simulink model "FlightSim"  (R2023a) (continued)
== system FlightSim/Aerodyanmic Forces/Axial Drag == (continued)
  bodyLength/1 -> CD(friction) calculation/1(bodyLength)
  finArea/1 -> CD(friction) calculation/2(finPlanformArea)
  finMACLength/1 -> CD(friction) calculation/3(MACLength)
  finThickness/1 -> CD(friction) calculation/6(finThickness), Body Pressure Drag/2(finThicknes)
  finCount/1 -> allFinCdFriciton/2, Body Pressure Drag/3(finCount)
  finProfileType/1 -> Body Pressure Drag/4(finProfileType)
  finSpan/1 -> Body Pressure Drag/5(finSpan)
  noseConeType/1 -> Body Pressure Drag/7(noseConeType)
  noseConeWettedArea/1 -> CD(friction) calculation/7(noseConeWettedArea)
  noseConeJoinAngle/1 -> Body Pressure Drag/8(noseConeJoinAngle)
  launchLugLength/1 -> Body Pressure Drag/9(launchLugLength)
  launchLugOuterDiameter/1 -> Body Pressure Drag/10(launchLugOuterDiameter)
  launchLugInnerDiameter/1 -> Body Pressure Drag/11(launchLugInnerDiameter)
  machNum/1 -> Body Pressure Drag/12(MachNum), SkinFrictionCoefficient/5(MachNum)
  AOA/1 -> CDtoAxailCD/2(AOA)
  finFrontSweepAngle/1 -> Body Pressure Drag/6(finFrontSweepAngle)
  SkinFrictionCoefficient/1(Cf) -> Display/1, CD(friction) calculation/4(Cf)
  rocketLength/1 -> SkinFrictionCoefficient/1(rocketLength)
  rocketVelocity/1 -> SkinFrictionCoefficient/2(rocketVelocity)
  airKinematicViscocity/1 -> SkinFrictionCoefficient/3(kinematic Viscosity)
  bodyR_S (roughness)/1 -> SkinFrictionCoefficient/4(R_s)
  CD(friction) calculation/3(Cd Friction Single Fin) -> allFinCdFriciton/1
  allFinCdFriciton/1 -> Add1/3, Display3/1
  noseConeHeight/1 -> CD(friction) calculation/8(noseConeHeight)
  CD(friction) calculation/2(Cd Friction Nosecone) -> Display1/1, Add1/2
  Add1/1 -> totalFrictionCD/1, Add/1

== system FlightSim/Aerodyanmic Forces/Canard Roll Calc ==
  note: FinSetCalc.Java file,line 184
  bodyDiameter : Inport
  finRootChord : Inport  Port=2
  finTailChord : Inport  Port=3
  finSpan : Inport  Port=4
  radialMACPosition : Inport  Port=5
  finCount : Inport  Port=6
  singleFinCNa : Inport  Port=7
  finCantAngle : Inport  Port=8
  " MachNum" : Inport  Port=9
  " rollRate" : Inport  Port=10
  " rocketVelocity" : Inport  Port=11
  Display : Display
  Display1 : Display
  Display2 : Display
  Product : Product
  RollDampeningCoefficient : Subsystem
  RollForcingCoefficient : Subsystem
  Subtract : Sum  Inputs=+-
  cRoll : Outport
  wires:
  bodyDiameter/1 -> RollDampeningCoefficient/3(bodyDiameter), RollForcingCoefficient/1(bodyDiameter)
  finRootChord/1 -> RollDampeningCoefficient/1(rootChord)
  finSpan/1 -> RollDampeningCoefficient/4(span), RollForcingCoefficient/5(finSpan)
  finCantAngle/1 -> RollForcingCoefficient/4(finCantAngle)
  radialMACPosition/1 -> RollForcingCoefficient/2(radialMACPosition)
  finTailChord/1 -> RollDampeningCoefficient/2(tailChord)
  finCount/1 -> Product/1
  singleFinCNa/1 -> RollForcingCoefficient/3(CNa)
  RollForcingCoefficient/1(singeFinRollForcing) -> Display/1, Subtract/1
  RollDampeningCoefficient/1(singleFinRollDamping) -> Display1/1, Subtract/2
  " MachNum"/1 -> RollDampeningCoefficient/5(MachNum)
  " rollRate"/1 -> RollDampeningCoefficient/6(rollRate)
  " rocketVelocity"/1 -> RollDampeningCoefficient/7(rocketVelocity)
  Subtract/1 -> Display2/1, Product/2
  Product/1 -> cRoll/1

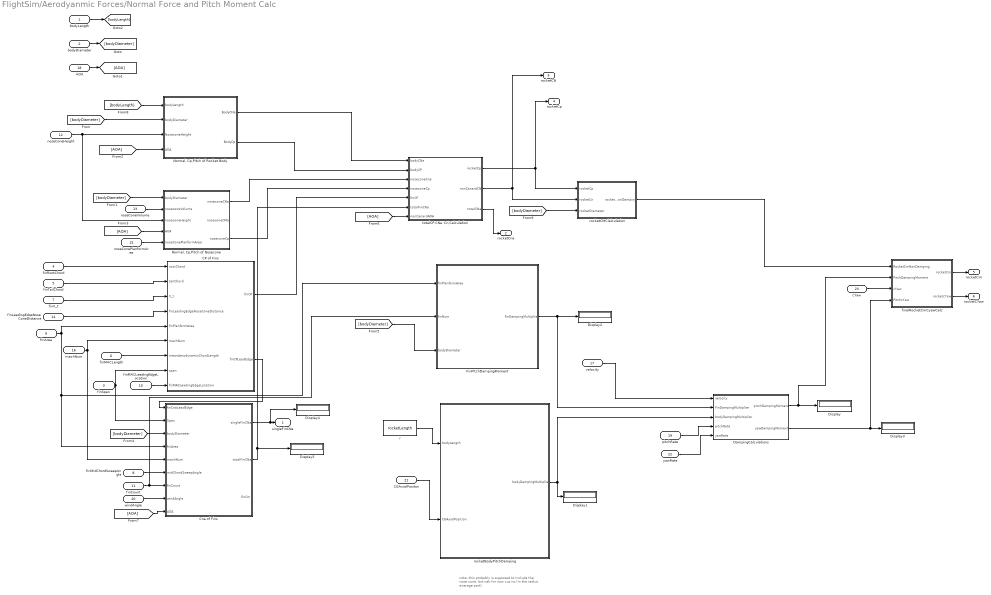
== system FlightSim/Aerodyanmic Forces/Normal Force and Pitch Moment Calc ==
  note: note: this probably is supposed to include the nose cone, but nah for now cuz no (in the radius average part)
  bodyLength : Inport
  bodyDiameter : Inport  Port=2
  finSpan : Inport  Port=3
  finRootChord : Inport  Port=4
  finTailChord : Inport  Port=5
  finMACLength : Inport  Port=6
  finX_t : Inport  Port=7
  finMidChordSweepAngle : Inport  Port=8
  finArea : Inport  Port=9
  finMACLeadingEdgeLocation : Inport  Port=10
  finCount : Inport  Port=11
  noseConeHeight : Inport  Port=12
  noseConeVolume : Inport  Port=13
  finLeadingEdgeNoseConeDistance : Inport  Port=14
  noseConePlanformArea : Inport  Port=15
  machNum : Inport  Port=16
  velocity : Inport  Port=17
  AOA : Inport  Port=18
  pitchRate : Inport  Port=19
  windAngle : Inport  Port=20
  CGAxialPositon : Inport  Port=21
  yawRate : Inport  Port=22
  cYaw : Inport  Port=23
  CP of Fins : Subsystem
  Cna of Fins : Subsystem
  DampingCalculations : Subsystem
  Display{,1..5} (6 alike) : Display
  FinPitchDampingMoment : Subsystem
  "Normal, Cp,Pitch of Nosecone" : Subsystem
  "Normal, Cp,Pitch of Rocket Body" : Subsystem
  finalRocketCmCyawCalc : Subsystem
  r : Constant  Value=rocketLength
  rocketBodyPitchDamping : Subsystem
  rocketCMClalculation : Subsystem
  "totalCP,CNa, Cn,Calculation" : Subsystem
  singleFinCNa : Outport
  rocketCna : Outport  Port=2
  rocketCN : Outport  Port=3
  rocketCp : Outport  Port=4
  rocketCm : Outport  Port=5
  rocketCYaw : Outport  Port=6
  wires:
  "Normal, Cp,Pitch of Rocket Body"/1(BodyCNa) -> "totalCP,CNa, Cn,Calculation"/1(bodyCNa)
  "Normal, Cp,Pitch of Rocket Body"/2(BodyCp) -> "totalCP,CNa, Cn,Calculation"/2(bodyCP)
  CP of Fins/1(finCP) -> "totalCP,CNa, Cn,Calculation"/5(finCP)
  "Normal, Cp,Pitch of Nosecone"/3(noseconeCp) -> "totalCP,CNa, Cn,Calculation"/4(noseconeCp)
  "Normal, Cp,Pitch of Nosecone"/1(noseconeCNa) -> "totalCP,CNa, Cn,Calculation"/3(noseconeCna)
  "totalCP,CNa, Cn,Calculation"/3(totalCNa) -> rocketCna/1
  "totalCP,CNa, Cn,Calculation"/1(rocketCp) -> rocketCMClalculation/1(rocketCp), rocketCp/1
  bodyDiameter/1 -> [bodyDiameter]
  [bodyDiameter] -> "Normal, Cp,Pitch of Rocket Body"/2(bodyDiameter)
  noseConeHeight/1 -> "Normal, Cp,Pitch of Rocket Body"/3(NoseconeHeight), "Normal, Cp,Pitch of Nosecone"/3(noseconeHeight)
  [bodyDiameter] -> "Normal, Cp,Pitch of Nosecone"/1(bodyDiameter)
  noseConeVolume/1 -> "Normal, Cp,Pitch of Nosecone"/2(noseconeVolume)
  AOA/1 -> [AOA]
  [AOA] -> "Normal, Cp,Pitch of Rocket Body"/4(AOA)
  [AOA] -> "Normal, Cp,Pitch of Nosecone"/4(AOA)
  finRootChord/1 -> CP of Fins/1(rootChord)
  finTailChord/1 -> CP of Fins/2(tailChord)
  finX_t/1 -> CP of Fins/3(X_t)
  finSpan/1 -> CP of Fins/8(span), Cna of Fins/2(Span)
  finMACLength/1 -> CP of Fins/7(meanAerodynamicChordLength)
  [bodyDiameter] -> Cna of Fins/3(bodyDiameter)
  finArea/1 -> CP of Fins/5(finPlanformArea), FinPitchDampingMoment/1(finPlanformArea), Cna of Fins/4(finArea)
  machNum/1 -> CP of Fins/6(machNum), Cna of Fins/5(machNum)
  finMidChordSweepAngle/1 -> Cna of Fins/6(midChordSweepAngle)
  finCount/1 -> Cna of Fins/7(finCount), FinPitchDampingMoment/2(finNum)
  finLeadingEdgeNoseConeDistance/1 -> CP of Fins/4(finLeadingEdgeNoseConeDistance)
  windAngle/1 -> Cna of Fins/8(windAngle)
  [bodyDiameter] -> FinPitchDampingMoment/3(bodyDiameter)
  [AOA] -> "totalCP,CNa, Cn,Calculation"/7(nonCanardAOA)
  Cna of Fins/2(totalFinCNa) -> Display5/1, "totalCP,CNa, Cn,Calculation"/6(totalFinCNa)
  Cna of Fins/1(singleFinCNa) -> Display4/1, singleFinCNa/1
  bodyLength/1 -> [bodyLength]
  [bodyLength] -> "Normal, Cp,Pitch of Rocket Body"/1(bodyLength)
  CGAxialPositon/1 -> rocketBodyPitchDamping/2(CGAxialPosition)
  rocketBodyPitchDamping/1(bodyDampingMultiplier) -> DampingCalculations/3(bodyDampingMultiplier), Display1/1
  "totalCP,CNa, Cn,Calculation"/2(nonCanardCN) -> rocketCMClalculation/2(rocketCn), rocketCN/1
  finMACLeadingEdgeLocation/1 -> CP of Fins/9(finMACLeadingEdgeLocation)
  noseConePlanformArea/1 -> "Normal, Cp,Pitch of Nosecone"/5(noseConePlanformArea)
  FinPitchDampingMoment/1(finDampingMultiplier) -> DampingCalculations/2(FinDampingMultiplier), Display2/1
  r/1 -> rocketBodyPitchDamping/1(bodyLength)
  yawRate/1 -> DampingCalculations/5(yawRate)
  DampingCalculations/1(pitchDampingMoment) -> finalRocketCmCyawCalc/2(PitchDampingMoment), Display/1
  DampingCalculations/2(yawDampingMoment) -> finalRocketCmCyawCalc/4(PitchcYaw), Display3/1
  velocity/1 -> DampingCalculations/1(velocity)
  pitchRate/1 -> DampingCalculations/4(pitchRate)
  CP of Fins/2(finCPLeadEdge) -> Cna of Fins/1(finCnaLeadEdge)
  [AOA] -> Cna of Fins/9(AOA)
  [bodyDiameter] -> rocketCMClalculation/3(rocketDiameter)
  cYaw/1 -> finalRocketCmCyawCalc/3(cYaw)
  rocketCMClalculation/1(rocketCmNonDamping) -> finalRocketCmCyawCalc/1(RocketCmNonDamping)
  finalRocketCmCyawCalc/1(rocketCm) -> rocketCm/1
  finalRocketCmCyawCalc/2(rocketcYaw) -> rocketCYaw/1

== system FlightSim/Forces and Rotations/CG Calculations ==
  note: translates from CP relative to nosecone to CP relative to base (need since CG relative to base)
  note: Rotating dCG/dt in body axis to dCG/dt in earth axis
  note: Adds dCG/dt to CG position, finding the new CG position in earth axis
  note: Distance between CP and Cg
  note: Convert Cp orentation from body axis to world axis
  note: Add's Cg so that Cp is now not relative to Cg but to world axis
  CGPosition : Inport
  rotationMatrix : Inport  Port=2
  rocketNonMotorMass : Inport  Port=3
  RocketCp : Inport  Port=4
  motorCasingMass : Inport  Port=5
  m_dot : Inport  Port=6
  motorPropMass : Inport  Port=7
  rocketLength : Inport  Port=8
  Add : Sum
  Add1 : Sum
  Body Frame CG : Subsystem
  Body Orientation : Product  Multiplication=Matrix(*)
  Body Orientation1 : Product  Multiplication=Matrix(*)
  Subtract : Sum  Inputs=+-
  Subtract1 : Sum  Inputs=+-
  AdjustedCG : Outport
  AdjustedCP : Outport  Port=2
  bodyFrameCg : Outport  Port=3
  wires:
  rocketNonMotorMass/1 -> Body Frame CG/1(rocketNonMotorMass)
  motorCasingMass/1 -> Body Frame CG/2(motorCasingMass)
  motorPropMass/1 -> Body Frame CG/3(motorPropMass)
  m_dot/1 -> Body Frame CG/4(m_dot)
  rotationMatrix/1 -> Body Orientation/1, Body Orientation1/1
  Body Frame CG/2(bodyFrameCG_dot) -> Body Orientation/2
  Body Orientation/1 -> Add/2
  Body Frame CG/1(bodyFrameCG) -> bodyFrameCg/1, Subtract1/2
  rocketLength/1 -> Subtract/1
  Add/1 -> AdjustedCG/1
  RocketCp/1 -> Subtract/2
  Subtract/1 -> Subtract1/1
  Subtract1/1 -> Body Orientation1/2
  CGPosition/1 -> Add1/2, Add/1
  Body Orientation1/1 -> Add1/1
  Add1/1 -> AdjustedCP/1

== system FlightSim/Forces and Rotations/Dynamic Pressure ==
  In1 : Inport
  In3 : Inport  Port=2
  In2 : Inport  Port=3
  Air density : Constant  Value=1.225
  Gain : Gain  Gain=1/2
  Mux1 : Mux  Inputs=3
  Product : Product
  Square : Math  Operator=square
  Transpose : Math  Operator=transpose
  Out1 : Outport
  wires:
  In1/1 -> Mux1/1
  Transpose/1 -> Out1/1
  Mux1/1 -> Square/1
  Air density/1 -> Product/2
  In3/1 -> Mux1/3
  Square/1 -> Product/1
  Product/1 -> Gain/1
  In2/1 -> Mux1/2
  Gain/1 -> Transpose/1

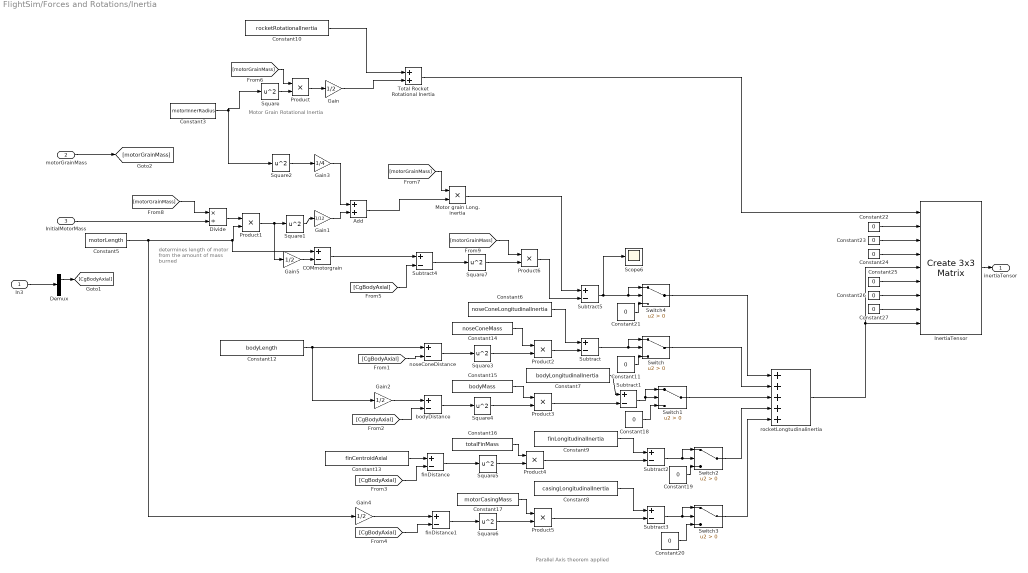
== system FlightSim/Forces and Rotations/Inertia ==
  note: Parallel Axis theorem applied
  note: Motor Grain Rotational Inertia
  note: determines length of motor from the amount of mass burned
  In3 : Inport
  motorGrainMass : Inport  Port=2
  InitialMotorMass : Inport  Port=3
  Add : Sum
  COMmotorgrain : Sum  Inputs=+-
  Constant10 : Constant  Value=rocketRotationalInertia
  Constant{11,18..27} (11 alike) : Constant  Value=0
  Constant12 : Constant  Value=bodyLength
  Constant13 : Constant  Value=finCentroidAxial
  Constant14 : Constant  Value=noseConeMass
  Constant15 : Constant  Value=bodyMass
  Constant16 : Constant  Value=totalFinMass
  Constant17 : Constant  Value=motorCasingMass
  Constant3 : Constant  Value=motorInnerRadius
  Constant5 : Constant  Value=motorLength
  Constant6 : Constant  Value=noseConeLongitudinalInertia
  Constant7 : Constant  Value=bodyLongitudinalInertia
  Constant8 : Constant  Value=casingLongitudinalInertia
  Constant9 : Constant  Value=finLongitudinalInertia
  Demux : Demux  Outputs=3
  Divide : Product  Inputs=*/
  Gain{,2,4..5} (4 alike) : Gain  Gain=1/2
  Gain1 : Gain  Gain=1/12
  Gain3 : Gain  Gain=1/4
  InertiaTensor : sharedutil/Create 3x3 Matrix  aMode=aMode
      settings: LibrarySourceBlock=aerolibutil/Create 3x3 Matrix
  Motor grain Long. inertia : Product
  Product{,1..6} (7 alike) : Product
  Scope6 : Scope
  Square{,1..7} (8 alike) : Math  Operator=square
  Subtract{,1..5} (6 alike) : Sum  Inputs=+-
  Switch{,1..4} (5 alike) : Switch  Criteria=u2 > Threshold  SaturateOnIntegerOverflow=off  InputSameDT=off
  Total Rocket Rotational Inertia : Sum
  bodyDistance : Sum  Inputs=+-
  finDistance : Sum  Inputs=+-
  finDistance1 : Sum  Inputs=+-
  noseConeDistance : Sum  Inputs=+-
  rocketLongtudinalinertia : Sum  Inputs=+++++
  inertiaTensor : Outport
  wires:
  In3/1 -> Demux/1
  Constant25/1 -> InertiaTensor/6
  [motorGrainMass] -> Product/1
  Motor grain Long. inertia/1 -> Subtract5/1
  motorGrainMass/1 -> [motorGrainMass]
  [motorGrainMass] -> Product6/1
  [motorGrainMass] -> Motor grain Long. inertia/1
  Square3/1 -> Product2/2
  Constant10/1 -> Total Rocket Rotational Inertia/1
  Square5/1 -> Product4/2
  Gain5/1 -> COMmotorgrain/2
  Gain3/1 -> Add/1
  Square6/1 -> Product5/2
  Constant7/1 -> Subtract1/1
  noseConeDistance/1 -> Square3/1
  Constant3/1 -> Square/1, Square2/1
  Square1/1 -> Gain1/1
  Product1/1 -> Gain5/1, Square1/1
  Constant22/1 -> InertiaTensor/2
  [CgBodyAxial] -> bodyDistance/2
  Subtract1/1 -> Switch1/2, Switch1/1
  [CgBodyAxial] -> finDistance1/2
  Subtract4/1 -> Square7/1
  Square4/1 -> Product3/2
  Subtract2/1 -> Switch2/1, Switch2/2
  Switch1/1 -> rocketLongtudinalinertia/3
  [CgBodyAxial] -> Subtract4/2
  Gain4/1 -> finDistance1/1
  Switch3/1 -> rocketLongtudinalinertia/5
  Constant17/1 -> Product5/1
  Product6/1 -> Subtract5/2
  finDistance1/1 -> Square6/1
  Switch4/1 -> rocketLongtudinalinertia/1
  rocketLongtudinalinertia/1 -> InertiaTensor/9, InertiaTensor/5
  Divide/1 -> Product1/1
  InertiaTensor/1 -> inertiaTensor/1
  Constant14/1 -> Product2/1
  Subtract3/1 -> Switch3/2, Switch3/1
  Constant26/1 -> InertiaTensor/7
  Constant5/1 -> COMmotorgrain/1, Product1/2, Gain4/1
  Switch2/1 -> rocketLongtudinalinertia/4
  Gain/1 -> Total Rocket Rotational Inertia/2
  Gain1/1 -> Add/2
  Square/1 -> Product/2
  Gain2/1 -> bodyDistance/1
  Constant13/1 -> finDistance/1
  Constant27/1 -> InertiaTensor/8
  finDistance/1 -> Square5/1
  Constant23/1 -> InertiaTensor/3
  Constant8/1 -> Subtract3/1
  Constant20/1 -> Switch3/3
  [CgBodyAxial] -> noseConeDistance/2
  [motorGrainMass] -> Divide/1
  Square2/1 -> Gain3/1
  Product2/1 -> Subtract/2
  Constant21/1 -> Switch4/3
  Total Rocket Rotational Inertia/1 -> InertiaTensor/1
  Subtract5/1 -> Scope6/1, Switch4/1, Switch4/2
  Constant16/1 -> Product4/1
  Product/1 -> Gain/1
  COMmotorgrain/1 -> Subtract4/1
  Switch/1 -> rocketLongtudinalinertia/2
  Product3/1 -> Subtract1/2
  Subtract/1 -> Switch/1, Switch/2
  InitialMotorMass/1 -> Divide/2
  Square7/1 -> Product6/2
  Add/1 -> Motor grain Long. inertia/2
  Constant24/1 -> InertiaTensor/4
  Constant15/1 -> Product3/1
  Constant19/1 -> Switch2/3
  Constant9/1 -> Subtract2/1
  Demux/1 -> [CgBodyAxial]
  Constant18/1 -> Switch1/3
  Product5/1 -> Subtract3/2
  Constant12/1 -> noseConeDistance/1, Gain2/1
  Constant6/1 -> Subtract/1
  Product4/1 -> Subtract2/2
  Constant11/1 -> Switch/3
  bodyDistance/1 -> Square4/1
  [CgBodyAxial] -> finDistance/2

== system FlightSim/Forces and Rotations/Thrust, m_dot Block ==
  note: lookup table will change depending on motor type (need to change manually)
  motorTotalImpulse : Inport
  motorInitialPropMass : Inport  Port=2
  In3 : Inport  Port=3
  Clock : Clock
  Divide : Product  Inputs=*/
  Gain : Gain  Gain=-1
  Integrator : Integrator  InitialConditionSource=external
  MotorMass : Scope
  Product : Product
  Scope2 : Scope
  Scope3 : Scope
  "Thrust Table, Aerotech G8" : Lookup_n-D  NumberOfTableDimensions=1  BreakpointsForDimension1=|  Table=|  InternalRulePriority=Speed  RndMeth=Simplest
      BreakpointsForDimension1=|
      | [0.024
      | 0.066
      | 0.138
      | 0.246
      | 0.426
      | 1.218
      | 2.082
      | 3.306
      | 5.322
      | 6.978
      | 8.632
      | 10.144
      | 12.088
      | 15.472
      | 17.632
      | 19.108
      | 19.511
      | 19.804
      | 19.867
      | 19.937
      | 19.964]
      Table=|
      | [0.3704
      | 0.8746
      | 4.5044
      | 6.6207
      | 6.7351
      | 7.3232
      | 7.423
      | 7.183
      | 6.8385
      | 6.7193
      | 6.5512
      | 6.4792
      | 6.4254
      | 6.3333
      | 6.1305
      | 6.0385
      | 5.9607
      | 4.4295
      | 1.6687
      | 0.3341
      | 0]
  dm/dt : Scope
  Thrust : Outport
  m_dot : Outport  Port=2
  motorPropMass : Outport  Port=3
  wires:
  motorTotalImpulse/1 -> Divide/2
  Clock/1 -> "Thrust Table, Aerotech G8"/1
  "Thrust Table, Aerotech G8"/1 -> Thrust/1, Scope2/1, Divide/1
  Gain/1 -> m_dot/1, dm/dt/1, Integrator/1
  motorInitialPropMass/1 -> Integrator/2, Product/1
  Integrator/1 -> motorPropMass/1, MotorMass/1
  Divide/1 -> Scope3/1, Product/2
  Product/1 -> Gain/1

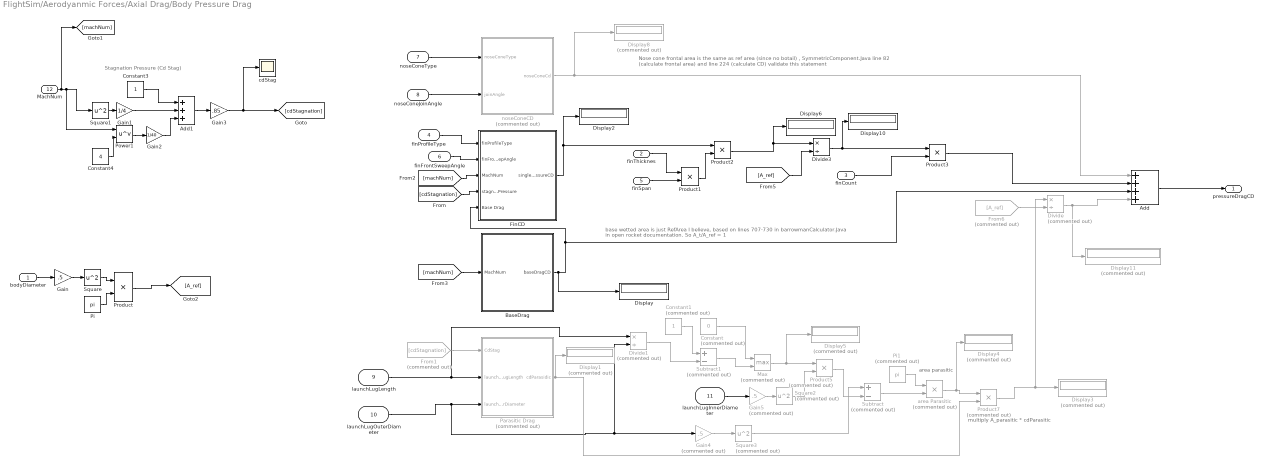
== system FlightSim/Aerodyanmic Forces/Axial Drag/Body Pressure Drag ==
  note: multiply A_parasitic * cdParasitic
  note: area parasitic
  note: base wetted area is just RefArea I believe, based on lines 707-730 in barrowmanCalculator.Java in open rocket documentation. So A_t/A_ref = 1
  note: Stagnation Pressure (Cd Stag)
  note: Nose cone frontal area is the same as ref area (since no botail) , SymmetricComponent.Java line 82 (calculate frontal area) and line 224 (calculate CD) validate this statement
  bodyDiameter : Inport
  finThicknes : Inport  Port=2
  finCount : Inport  Port=3
  finProfileType : Inport  Port=4
  finSpan : Inport  Port=5
  finFrontSweepAngle : Inport  Port=6
  noseConeType : Inport  Port=7
  noseConeJoinAngle : Inport  Port=8
  launchLugLength : Inport  Port=9
  launchLugOuterDiameter : Inport  Port=10
  launchLugInnerDiameter : Inport  Port=11
  MachNum : Inport  Port=12
  Add : Sum  Inputs=++++
  Add1 : Sum  Inputs=+++
  BaseDrag : Subsystem
  Constant : Constant  Commented=on  Value=0
  Constant1 : Constant  Commented=on
  Constant3 : Constant
  Constant4 : Constant  Value=4
  Display{,2,6,10} (4 alike) : Display
  Display{1,3..5,8,11} (6 alike) : Display  Commented=on
  Divide : Product  Commented=on  Inputs=*/
  Divide1 : Product  Commented=on  Inputs=*/
  Divide3 : Product  Inputs=*/
  FinCD : Subsystem
  From1 : From  Commented=on  GotoTag=cdStagnation
  From6 : From  Commented=on  GotoTag=A_ref
  Gain : Gain  Gain=.5
  Gain1 : Gain  Gain=1/4
  Gain2 : Gain  Gain=1/40
  Gain3 : Gain  Gain=.85
  Gain4 : Gain  Commented=on  Gain=.5
  Gain5 : Gain  Commented=on  Gain=.5
  Max : MinMax  Commented=on  Function=max  Inputs=2
  Parasitic Drag : Subsystem  Commented=on
  Pi : Constant  Value=pi  OutDataTypeStr=Inherit: Inherit via back propagation
  Pi1 : Constant  Commented=on  Value=pi  OutDataTypeStr=Inherit: Inherit via back propagation
  Power1 : Math  Operator=pow
  Product{,1..3} (4 alike) : Product
  Product5 : Product  Commented=on
  Product7 : Product  Commented=on
  Square : Math  Operator=square
  Square1 : Math  Operator=square
  Square2 : Math  Commented=on  Operator=square
  Square3 : Math  Commented=on  Operator=square
  Subtract : Sum  Commented=on  Inputs=+-
  Subtract1 : Sum  Commented=on  Inputs=+-
  area Parasitic : Product  Commented=on
  cdStag : Scope
  noseConeCD : Subsystem  Commented=on
  pressureDragCD : Outport
  wires:
  BaseDrag/1(baseDragCD) -> Display/1, Add/3, FinCD/5("Base Drag ")
  finThicknes/1 -> Product1/1
  finSpan/1 -> Product1/2
  finCount/1 -> Product3/2
  FinCD/1(singleFinPressureCD) -> Display2/1, Product2/1
  Product1/1 -> Product2/2
  Product2/1 -> Divide3/1, Display6/1
  Gain2/1 -> Add1/3
  Constant3/1 -> Add1/1
  Gain1/1 -> Add1/2
  MachNum/1 -> [machNum], Power1/1, Square1/1
  Constant4/1 -> Power1/2
  Square1/1 -> Gain1/1
  Power1/1 -> Gain2/1
  Add1/1 -> Gain3/1
  launchLugLength/1 -> Divide1/1, Parasitic Drag/2(launchLugLength)
  launchLugOuterDiameter/1 -> Divide1/2, Gain4/1, Parasitic Drag/3(launchLugOuterDiameter)
  Constant1/1 -> Subtract1/1
  Divide1/1 -> Subtract1/2
  Subtract1/1 -> Max/2
  Constant/1 -> Max/1
  Max/1 -> Display5/1, Product5/1
  Gain5/1 -> Square2/1
  launchLugInnerDiameter/1 -> Gain5/1
  Square2/1 -> Product5/2
  Product5/1 -> Subtract/2
  Square3/1 -> Subtract/1
  Pi1/1 -> area Parasitic/1
  Subtract/1 -> area Parasitic/2
  Parasitic Drag/1(cdParasidic) -> Product7/2, Display1/1
  area Parasitic/1 -> Display4/1, Product7/1
  Product7/1 -> Divide/1, Display3/1
  Gain4/1 -> Square3/1
  Gain3/1 -> [cdStagnation], cdStag/1
  [cdStagnation] -> FinCD/4(stagnationPressure)
  From1/1 -> Parasitic Drag/1(CdStag)
  noseConeType/1 -> noseConeCD/1(noseConeType)
  noseConeJoinAngle/1 -> noseConeCD/2(joinAngle)
  finProfileType/1 -> FinCD/1(finProfileType)
  [machNum] -> FinCD/3(MachNum)
  [machNum] -> BaseDrag/1(MachNum)
  Square/1 -> Product/1
  Pi/1 -> Product/2
  Gain/1 -> Square/1
  bodyDiameter/1 -> Gain/1
  [A_ref] -> Divide3/2
  From6/1 -> Divide/2
  Add/1 -> pressureDragCD/1
  Divide/1 -> Display11/1, Add/4
  Divide3/1 -> Product3/1, Display10/1
  noseConeCD/1(noseConeCd) -> Display8/1, Add/1
  Product/1 -> [A_ref]
  finFrontSweepAngle/1 -> FinCD/2(finFrontSweepAngle)
  Product3/1 -> Add/2

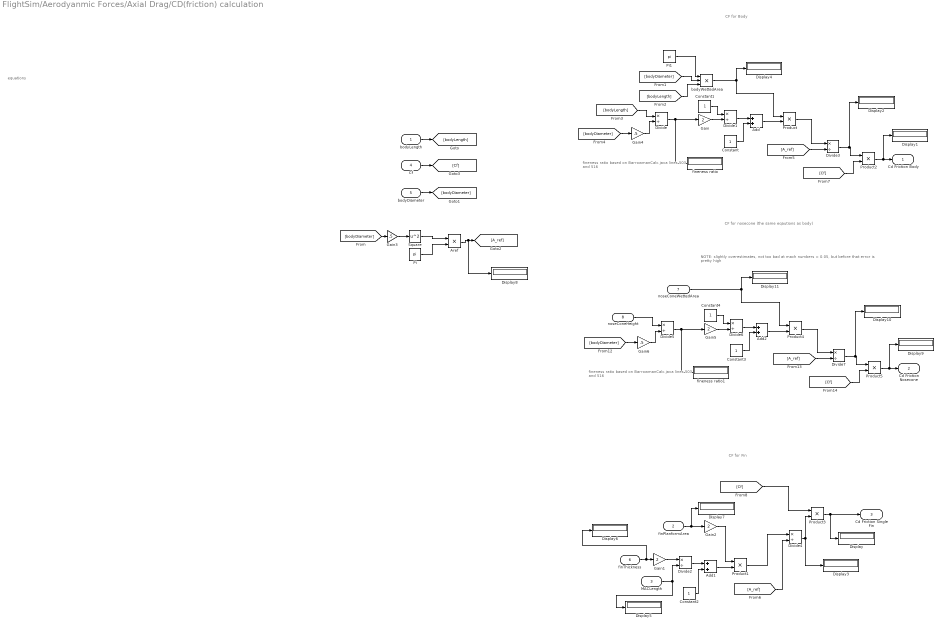
== system FlightSim/Aerodyanmic Forces/Axial Drag/CD(friction) calculation ==
  note: NOTE: slightly overestimates, not too bad at mach numbers > 0.05, but before that error is pretty high
  note: CF for Body
  note: fineness ratio based on BarrowmanCalc.java lines
      |  503 and 516
  note: equations
  note: CF for Fin
  note: CF for nosecone (the same eqautions as body)
  note: fineness ratio based on BarrowmanCalc.java lines
      |  503 and 516
  bodyLength : Inport
  finPlanformArea : Inport  Port=2
  MACLength : Inport  Port=3
  Cf : Inport  Port=4
  bodyDiameter : Inport  Port=5
  finThickness : Inport  Port=6
  noseConeWettedArea : Inport  Port=7
  noseConeHeight : Inport  Port=8
  Add : Sum
  Add1 : Sum
  Add2 : Sum
  Aref : Product
  Constant{,1..4} (5 alike) : Constant
  Display{,1..11} (12 alike) : Display
  Divide{,1..7} (8 alike) : Product  Inputs=*/
  Gain{,1..2,5} (4 alike) : Gain  Gain=2
  Gain3 : Gain  Gain=.5
  Gain4 : Gain  Gain=.5
  Gain6 : Gain  Gain=.5
  Pi : Constant  Value=pi  OutDataTypeStr=Inherit: Inherit via back propagation
  Pi1 : Constant  Value=pi  OutDataTypeStr=Inherit: Inherit via back propagation
  Product{,1..5} (6 alike) : Product
  Square : Math  Operator=square
  bodyWettedArea : Product  Inputs=3
  fineness ratio : Display
  fineness ratio1 : Display
  Cd Friction Body : Outport
  Cd Friction Nosecone : Outport  Port=2
  Cd Friction Single Fin : Outport  Port=3
  wires:
  Constant/1 -> Add/2
  Constant1/1 -> Divide1/1
  Divide1/1 -> Add/1
  Add/1 -> Product/2
  bodyWettedArea/1 -> Display4/1, Product/1
  Gain/1 -> Divide1/2
  finThickness/1 -> Display6/1, Gain1/1
  Gain1/1 -> Divide2/1
  MACLength/1 -> Display5/1, Divide2/2
  Constant2/1 -> Add1/2
  Divide2/1 -> Add1/1
  Add1/1 -> Product1/2
  Product/1 -> Divide3/1
  Product1/1 -> Divide4/1
  Square/1 -> Aref/1
  Pi/1 -> Aref/2
  Product2/1 -> Display1/1, Cd Friction Body/1
  finPlanformArea/1 -> Display7/1, Gain2/1
  Gain2/1 -> Product1/1
  bodyLength/1 -> [bodyLength]
  bodyDiameter/1 -> [bodyDiameter]
  [bodyDiameter] -> Gain3/1
  Gain3/1 -> Square/1
  Aref/1 -> Display8/1, [A_ref]
  [bodyDiameter] -> bodyWettedArea/2
  [bodyLength] -> bodyWettedArea/3
  [bodyLength] -> Divide/1
  Divide/1 -> Gain/1, fineness ratio/1
  [A_ref] -> Divide3/2
  Divide3/1 -> Display2/1, Product2/1
  [A_ref] -> Divide4/2
  Divide4/1 -> Product3/2, Display3/1
  Product3/1 -> Display/1, Cd Friction Single Fin/1
  [Cf] -> Product3/1
  Pi1/1 -> bodyWettedArea/1
  [bodyDiameter] -> Gain4/1
  Gain4/1 -> Divide/2
  Cf/1 -> [Cf]
  [Cf] -> Product2/2
  [Cf] -> Product5/2
  Product5/1 -> Display9/1, Cd Friction Nosecone/1
  Add2/1 -> Product4/2
  Constant4/1 -> Divide6/1
  Gain6/1 -> Divide5/2
  noseConeHeight/1 -> Divide5/1
  Product4/1 -> Divide7/1
  Constant3/1 -> Add2/2
  Gain5/1 -> Divide6/2
  Divide5/1 -> Gain5/1, fineness ratio1/1
  Divide6/1 -> Add2/1
  [bodyDiameter] -> Gain6/1
  Divide7/1 -> Display10/1, Product5/1
  [A_ref] -> Divide7/2
  noseConeWettedArea/1 -> Product4/1, Display11/1

== system FlightSim/Aerodyanmic Forces/Axial Drag/CDtoAxailCD ==
  note: Segment from open Rocket Document
  note: equations
  note: Equation for CD drag Multiplier between 0-17 degrees (in radians ). This was derivated from the Open Rocket Source Code. Source code did not directly provide ceofficients, so the code related to calculating coefficients was placed into a separate file outside of Open rocket's docuementation and ran to find the coefficents
  note: Equation for CD drag Multiplier between 17-90 degrees (in radians ). Derivated in the same way as Previous equation
  CD : Inport
  AOA : Inport  Port=2
  Display : Display
  Polynomial : Polyval  Coefs="[ -1.480006750190687,  6.784940235048049,-10.062655893223182,  4.334008218595031, 0.7341792753994868]"
  Polynomial1 : Polyval  Coefs=[ -22.970602338418814, 10.223272370278785,0,1]
  Product : Product
  Switch : Switch  Criteria=u2 > Threshold  Threshold=17*pi/180  SaturateOnIntegerOverflow=off  InputSameDT=off
  axialCD : Outport
  wires:
  CD/1 -> Product/1
  AOA/1 -> Polynomial1/1, Polynomial/1, Switch/2
  Product/1 -> axialCD/1
  Polynomial/1 -> Switch/1
  Polynomial1/1 -> Switch/3
  Switch/1 -> Display/1, Product/2
